simulink model "CDF_VSVPWM_Grid_Parallel"  (R2024b) (continued)
== system CDF_VSVPWM_Grid_Parallel/VSVPWM1/矢量作用时间计算/I3 ==
  note: Ta
  note: Tb
  note: Tc
  k : Inport
  Ts : Inport  Port=2
  theta : Inport  Port=3
  Constant : Constant
  Constant1 : Constant
  Gain : Gain  Gain=sqrt(3)
  Gain1 : Gain  Gain=2
  Gain3 : Gain  Gain=3
  Mux : Mux  Inputs=3
  Product{,1..5} (6 alike) : Product
  Scope{,1..3} (4 alike) : Scope
  Sin1 : Trigonometry  Operator=cos
  Sin2 : Trigonometry
  Sum2 : Sum  Inputs=|+-
  Sum3 : Sum  Inputs=|-+
  sin(pi/6+u) : MATLAB Function  TreatAsAtomicUnit=on
  T : Outport
  wires:
  Product5/1 -> Product2/1
  Gain3/1 -> Product5/2
  Sin2/1 -> Product5/1
  Sum3/1 -> Product1/1
  Constant1/1 -> Sum3/1
  Product4/1 -> Sum3/2
  Sin1/1 -> Product4/2
  Ts/1 -> Product/2, Product1/2, Product2/2
  Gain1/1 -> Product/1
  Sum2/1 -> Gain1/1
  Constant/1 -> Sum2/1
  Product3/1 -> Sum2/2
  sin(pi/6+u)/1(y) -> Product3/2
  Gain/1 -> Product3/1, Product4/1
  k/1 -> Gain/1, Gain3/1
  theta/1 -> Sin2/1, sin(pi/6+u)/1(u), Sin1/1
  Product1/1 -> Scope1/1, Mux/2
  Product/1 -> Scope/1, Mux/1
  Mux/1 -> Scope3/1, T/1
  Product2/1 -> Mux/3, Scope2/1

== system CDF_VSVPWM_Grid_Parallel/VSVPWM1/矢量作用时间计算/I4 ==
  note: Ta
  note: Tb
  note: Tc
  k : Inport
  Ts : Inport  Port=2
  theta : Inport  Port=3
  Constant : Constant
  Constant1 : Constant
  Gain : Gain  Gain=sqrt(3)
  Gain1 : Gain  Gain=2
  Gain3 : Gain  Gain=3
  Mux : Mux  Inputs=3
  Product{,1..5} (6 alike) : Product
  Scope{,1..3} (4 alike) : Scope
  Sin1 : Trigonometry  Operator=cos
  Sum2 : Sum  Inputs=|+-
  Sum3 : Sum  Inputs=|+-
  sin(pi/3-u) : MATLAB Function  TreatAsAtomicUnit=on
  sin(pi/6+u) : MATLAB Function  TreatAsAtomicUnit=on
  T : Outport
  wires:
  Sum3/1 -> Product1/1
  Gain1/1 -> Product/1
  Sum2/1 -> Gain1/1
  Constant/1 -> Sum2/1
  Product3/1 -> Sum2/2
  Gain/1 -> Product4/1, Product3/1
  k/1 -> Gain/1, Gain3/1
  theta/1 -> sin(pi/3-u)/1(u), sin(pi/6+u)/1(u), Sin1/1
  Product1/1 -> Scope2/1, Mux/2
  Product/1 -> Scope1/1, Mux/1
  Mux/1 -> Scope/1, T/1
  Product2/1 -> Mux/3, Scope3/1
  Sin1/1 -> Product3/2
  Ts/1 -> Product2/2, Product1/2, Product/2
  sin(pi/6+u)/1(y) -> Product4/2
  Constant1/1 -> Sum3/2
  Product4/1 -> Sum3/1
  sin(pi/3-u)/1(y) -> Product5/2
  Gain3/1 -> Product5/1
  Product5/1 -> Product2/1

== system CDF_VSVPWM_Grid_Parallel/VSVPWM1/矢量作用时间计算/I5 ==
  note: Tc
  note: Tb
  note: Ta
  k : Inport
  Ts : Inport  Port=2
  theta : Inport  Port=3
  Constant : Constant
  Constant1 : Constant
  Gain : Gain  Gain=sqrt(3)
  Gain1 : Gain  Gain=3
  Mux : Mux  Inputs=3
  Product{,1..5} (6 alike) : Product
  Scope : Scope
  Scope1 : Scope
  Scope2 : Scope
  Sin1 : Trigonometry  Operator=cos
  Sum1 : Sum  Inputs=|+-
  Sum2 : Sum  Inputs=|-+
  Sum3 : Sum  Inputs=|+-
  sin(pi/3+u) : MATLAB Function  TreatAsAtomicUnit=on
  sin(pi/6+u) : MATLAB Function  TreatAsAtomicUnit=on
  T : Outport
  wires:
  k/1 -> Product5/1, Gain/1
  sin(pi/3+u)/1(y) -> Product5/2
  Product4/1 -> Sum3/1
  Constant1/1 -> Sum1/1, Sum3/2
  sin(pi/6+u)/1(y) -> Product4/2
  Ts/1 -> Product/2, Product1/2, Product2/2
  Sin1/1 -> Product3/2
  Mux/1 -> T/1
  Product/1 -> Mux/1, Scope/1
  Product1/1 -> Scope1/1, Mux/2
  theta/1 -> Sin1/1, sin(pi/6+u)/1(u), sin(pi/3+u)/1(u)
  Gain/1 -> Product3/1, Product4/1
  Product3/1 -> Sum2/2
  Constant/1 -> Sum2/1
  Sum2/1 -> Product/1
  Sum3/1 -> Product1/1
  Product5/1 -> Sum1/2
  Sum1/1 -> Product2/1
  Product2/1 -> Gain1/1
  Gain1/1 -> Mux/3, Scope2/1

== system CDF_VSVPWM_Grid_Parallel/VSVPWM/生成时间调制波/Ⅰ扇区的调制信号计算/1-1 ==
  time : Inport
  Demux : Demux  Outputs=5
  MATLAB Function : MATLAB Function  TreatAsAtomicUnit=on
  MATLAB Function1 : MATLAB Function  TreatAsAtomicUnit=on
  MATLAB Function2 : MATLAB Function  TreatAsAtomicUnit=on
  Mux : Mux  Inputs=6
  Scope : Scope
  Tabc12 : Outport
  wires:
  MATLAB Function/1(ta1) -> Mux/1
  time/1 -> Demux/1
  Mux/1 -> Scope/1, Tabc12/1
  Demux/1 -> MATLAB Function2/1(unused), MATLAB Function1/1(T1), MATLAB Function/1(T1)
  MATLAB Function/2(ta2) -> Mux/2
  MATLAB Function1/1(tb1) -> Mux/3
  MATLAB Function1/2(tb2) -> Mux/4
  MATLAB Function2/1(tc1) -> Mux/5
  MATLAB Function2/2(tc2) -> Mux/6
  Demux/2 -> MATLAB Function2/2(unused1), MATLAB Function1/2(unused), MATLAB Function/2(T2)
  Demux/3 -> MATLAB Function2/3(unused2), MATLAB Function1/3(unused1), MATLAB Function/3(unused)
  Demux/4 -> MATLAB Function2/4(T4), MATLAB Function1/4(unused2), MATLAB Function/4(unused1)
  Demux/5 -> MATLAB Function2/5(T5), MATLAB Function1/5(T5), MATLAB Function/5(unused2)

== system CDF_VSVPWM_Grid_Parallel/VSVPWM/生成时间调制波/Ⅰ扇区的调制信号计算/1-2 ==
  time : Inport
  Demux : Demux  Outputs=5
  MATLAB Function : MATLAB Function  TreatAsAtomicUnit=on
  MATLAB Function1 : MATLAB Function  TreatAsAtomicUnit=on
  MATLAB Function2 : MATLAB Function  TreatAsAtomicUnit=on
  Mux : Mux  Inputs=6
  Tabc12 : Outport
  wires:
  MATLAB Function2/2(tc2) -> Mux/6
  MATLAB Function2/1(tc1) -> Mux/5
  MATLAB Function1/2(tb2) -> Mux/4
  MATLAB Function1/1(tb1) -> Mux/3
  MATLAB Function/2(ta2) -> Mux/2
  Demux/5 -> MATLAB Function/5(unused1), MATLAB Function1/5(T5), MATLAB Function2/5(T5)
  Demux/4 -> MATLAB Function/4(unused), MATLAB Function1/4(unused2), MATLAB Function2/4(T4)
  Demux/2 -> MATLAB Function/2(T2), MATLAB Function1/2(unused), MATLAB Function2/2(unused1)
  Demux/1 -> MATLAB Function/1(T1), MATLAB Function1/1(T1), MATLAB Function2/1(unused)
  Mux/1 -> Tabc12/1
  Demux/3 -> MATLAB Function/3(T3), MATLAB Function1/3(unused1), MATLAB Function2/3(T3)
  time/1 -> Demux/1
  MATLAB Function/1(ta1) -> Mux/1

== system CDF_VSVPWM_Grid_Parallel/VSVPWM/生成时间调制波/Ⅰ扇区的调制信号计算/1-3 ==
  time : Inport
  Demux : Demux  Outputs=5
  MATLAB Function : MATLAB Function  TreatAsAtomicUnit=on
  MATLAB Function1 : MATLAB Function  TreatAsAtomicUnit=on
  MATLAB Function2 : MATLAB Function  TreatAsAtomicUnit=on
  Mux : Mux  Inputs=6
  Tabc12 : Outport
  wires:
  MATLAB Function/1(ta1) -> Mux/1
  time/1 -> Demux/1
  Demux/3 -> MATLAB Function2/3(T3), MATLAB Function1/3(unused1), MATLAB Function/3(T3)
  Mux/1 -> Tabc12/1
  Demux/1 -> MATLAB Function2/1(unused), MATLAB Function1/1(T1), MATLAB Function/1(T1)
  Demux/2 -> MATLAB Function2/2(unused1), MATLAB Function1/2(unused), MATLAB Function/2(T2)
  Demux/4 -> MATLAB Function2/4(T4), MATLAB Function1/4(T4), MATLAB Function/4(T4)
  Demux/5 -> MATLAB Function2/5(T5), MATLAB Function1/5(T5), MATLAB Function/5(unused)
  MATLAB Function/2(ta2) -> Mux/2
  MATLAB Function1/1(tb1) -> Mux/3
  MATLAB Function1/2(tb2) -> Mux/4
  MATLAB Function2/1(tc1) -> Mux/5
  MATLAB Function2/2(tc2) -> Mux/6

== system CDF_VSVPWM_Grid_Parallel/VSVPWM/生成时间调制波/Ⅰ扇区的调制信号计算/1-4 ==
  time : Inport
  Demux : Demux  Outputs=5
  MATLAB Function : MATLAB Function  TreatAsAtomicUnit=on
  MATLAB Function1 : MATLAB Function  TreatAsAtomicUnit=on
  MATLAB Function2 : MATLAB Function  TreatAsAtomicUnit=on
  Mux : Mux  Inputs=6
  Tabc12 : Outport
  wires:
  MATLAB Function2/2(tc2) -> Mux/6
  MATLAB Function2/1(tc1) -> Mux/5
  MATLAB Function1/2(tb2) -> Mux/4
  MATLAB Function1/1(tb1) -> Mux/3
  MATLAB Function/2(ta2) -> Mux/2
  Demux/5 -> MATLAB Function/5(unused1), MATLAB Function1/5(T5), MATLAB Function2/5(T5)
  Demux/4 -> MATLAB Function/4(unused), MATLAB Function1/4(unused1), MATLAB Function2/4(T4)
  Demux/2 -> MATLAB Function/2(T2), MATLAB Function1/2(T2), MATLAB Function2/2(T2)
  Demux/1 -> MATLAB Function/1(T1), MATLAB Function1/1(T1), MATLAB Function2/1(unused)
  Mux/1 -> Tabc12/1
  Demux/3 -> MATLAB Function/3(T3), MATLAB Function1/3(unused), MATLAB Function2/3(T3)
  time/1 -> Demux/1
  MATLAB Function/1(ta1) -> Mux/1

== system CDF_VSVPWM_Grid_Parallel/VSVPWM/生成时间调制波/Ⅰ扇区的调制信号计算/1-5 ==
  time : Inport
  Demux : Demux  Outputs=5
  MATLAB Function : MATLAB Function  TreatAsAtomicUnit=on
  MATLAB Function1 : MATLAB Function  TreatAsAtomicUnit=on
  MATLAB Function2 : MATLAB Function  TreatAsAtomicUnit=on
  Mux : Mux  Inputs=6
  Tabc12 : Outport
  wires:
  MATLAB Function/1(ta1) -> Mux/1
  time/1 -> Demux/1
  Demux/3 -> MATLAB Function2/3(T3), MATLAB Function1/3(unused), MATLAB Function/3(T3)
  Mux/1 -> Tabc12/1
  Demux/1 -> MATLAB Function2/1(unused), MATLAB Function1/1(T1), MATLAB Function/1(T1)
  Demux/2 -> MATLAB Function2/2(T2), MATLAB Function1/2(T2), MATLAB Function/2(T2)
  Demux/4 -> MATLAB Function2/4(T4), MATLAB Function1/4(T4), MATLAB Function/4(T4)
  Demux/5 -> MATLAB Function2/5(T5), MATLAB Function1/5(T5), MATLAB Function/5(unused)
  MATLAB Function/2(ta2) -> Mux/2
  MATLAB Function1/1(tb1) -> Mux/3
  MATLAB Function1/2(tb2) -> Mux/4
  MATLAB Function2/1(tc1) -> Mux/5
  MATLAB Function2/2(tc2) -> Mux/6

== system CDF_VSVPWM_Grid_Parallel/VSVPWM/生成时间调制波/Ⅱ扇区的调制信号计算/2-1 ==
  time : Inport
  Demux : Demux  Outputs=5
  MATLAB Function : MATLAB Function  TreatAsAtomicUnit=on
  MATLAB Function1 : MATLAB Function  TreatAsAtomicUnit=on
  MATLAB Function2 : MATLAB Function  TreatAsAtomicUnit=on
  Mux : Mux  Inputs=6
  Scope : Scope  NumInputPorts=6
  Scope1 : Scope
  Tabc12 : Outport
  wires:
  MATLAB Function2/2(tc2) -> Scope/6, Mux/6
  MATLAB Function2/1(tc1) -> Scope/5, Mux/5
  MATLAB Function1/2(tb2) -> Scope/4, Mux/4
  MATLAB Function1/1(tb1) -> Scope/3, Mux/3
  MATLAB Function/2(ta2) -> Mux/2, Scope/2
  Demux/5 -> MATLAB Function/5(T5), MATLAB Function1/5(unused2), MATLAB Function2/5(T5)
  Demux/4 -> MATLAB Function/4(unused2), MATLAB Function1/4(unused1), MATLAB Function2/4(T4)
  Demux/2 -> MATLAB Function/2(unused), MATLAB Function1/2(T2), MATLAB Function2/2(unused1)
  Demux/1 -> MATLAB Function/1(T1), MATLAB Function1/1(T1), MATLAB Function2/1(unused)
  Mux/1 -> Scope1/1, Tabc12/1
  Demux/3 -> MATLAB Function/3(unused1), MATLAB Function1/3(unused), MATLAB Function2/3(unused2)
  time/1 -> Demux/1
  MATLAB Function/1(ta1) -> Scope/1, Mux/1

== system CDF_VSVPWM_Grid_Parallel/VSVPWM/生成时间调制波/Ⅱ扇区的调制信号计算/2-2 ==
  time : Inport
  Demux : Demux  Outputs=5
  MATLAB Function : MATLAB Function  TreatAsAtomicUnit=on
  MATLAB Function1 : MATLAB Function  TreatAsAtomicUnit=on
  MATLAB Function2 : MATLAB Function  TreatAsAtomicUnit=on
  Mux : Mux  Inputs=6
  Tabc12 : Outport
  wires:
  MATLAB Function/1(ta1) -> Mux/1
  time/1 -> Demux/1
  Demux/3 -> MATLAB Function2/3(T3), MATLAB Function1/3(T3), MATLAB Function/3(unused1)
  Mux/1 -> Tabc12/1
  Demux/1 -> MATLAB Function2/1(unused), MATLAB Function1/1(T1), MATLAB Function/1(T1)
  Demux/2 -> MATLAB Function2/2(unused1), MATLAB Function1/2(T2), MATLAB Function/2(unused)
  Demux/4 -> MATLAB Function2/4(T4), MATLAB Function1/4(unused), MATLAB Function/4(unused2)
  Demux/5 -> MATLAB Function2/5(T5), MATLAB Function1/5(unused1), MATLAB Function/5(T5)
  MATLAB Function/2(ta2) -> Mux/2
  MATLAB Function1/1(tb1) -> Mux/3
  MATLAB Function1/2(tb2) -> Mux/4
  MATLAB Function2/1(tc1) -> Mux/5
  MATLAB Function2/2(tc2) -> Mux/6

== system CDF_VSVPWM_Grid_Parallel/VSVPWM/生成时间调制波/Ⅱ扇区的调制信号计算/2-3 ==
  time : Inport
  Demux : Demux  Outputs=5
  MATLAB Function : MATLAB Function  TreatAsAtomicUnit=on
  MATLAB Function1 : MATLAB Function  TreatAsAtomicUnit=on
  MATLAB Function2 : MATLAB Function  TreatAsAtomicUnit=on
  Mux : Mux  Inputs=6
  Tabc12 : Outport
  wires:
  MATLAB Function2/2(tc2) -> Mux/6
  MATLAB Function2/1(tc1) -> Mux/5
  MATLAB Function1/2(tb2) -> Mux/4
  MATLAB Function1/1(tb1) -> Mux/3
  MATLAB Function/2(ta2) -> Mux/2
  Demux/5 -> MATLAB Function/5(T5), MATLAB Function1/5(unused1), MATLAB Function2/5(T5)
  Demux/4 -> MATLAB Function/4(unused1), MATLAB Function1/4(unused), MATLAB Function2/4(T4)
  Demux/2 -> MATLAB Function/2(T2), MATLAB Function1/2(T2), MATLAB Function2/2(T2)
  Demux/1 -> MATLAB Function/1(T1), MATLAB Function1/1(T1), MATLAB Function2/1(unused)
  Mux/1 -> Tabc12/1
  Demux/3 -> MATLAB Function/3(unused), MATLAB Function1/3(T3), MATLAB Function2/3(T3)
  time/1 -> Demux/1
  MATLAB Function/1(ta1) -> Mux/1

== system CDF_VSVPWM_Grid_Parallel/VSVPWM/生成时间调制波/Ⅱ扇区的调制信号计算/2-4 ==
  time : Inport
  Demux : Demux  Outputs=5
  MATLAB Function : MATLAB Function  TreatAsAtomicUnit=on
  MATLAB Function1 : MATLAB Function  TreatAsAtomicUnit=on
  MATLAB Function2 : MATLAB Function  TreatAsAtomicUnit=on
  Mux : Mux  Inputs=6
  Tabc12 : Outport
  wires:
  MATLAB Function/1(ta1) -> Mux/1
  time/1 -> Demux/1
  Demux/3 -> MATLAB Function2/3(T3), MATLAB Function1/3(T3), MATLAB Function/3(unused1)
  Mux/1 -> Tabc12/1
  Demux/1 -> MATLAB Function2/1(unused), MATLAB Function1/1(T1), MATLAB Function/1(T1)
  Demux/2 -> MATLAB Function2/2(unused1), MATLAB Function1/2(T2), MATLAB Function/2(unused)
  Demux/4 -> MATLAB Function2/4(T4), MATLAB Function1/4(T4), MATLAB Function/4(T4)
  Demux/5 -> MATLAB Function2/5(T5), MATLAB Function1/5(unused), MATLAB Function/5(T5)
  MATLAB Function/2(ta2) -> Mux/2
  MATLAB Function1/1(tb1) -> Mux/3
  MATLAB Function1/2(tb2) -> Mux/4
  MATLAB Function2/1(tc1) -> Mux/5
  MATLAB Function2/2(tc2) -> Mux/6

== system CDF_VSVPWM_Grid_Parallel/VSVPWM/生成时间调制波/Ⅱ扇区的调制信号计算/2-5 ==
  time : Inport
  Demux : Demux  Outputs=5
  MATLAB Function : MATLAB Function  TreatAsAtomicUnit=on
  MATLAB Function1 : MATLAB Function  TreatAsAtomicUnit=on
  MATLAB Function2 : MATLAB Function  TreatAsAtomicUnit=on
  Mux : Mux  Inputs=6
  Tabc12 : Outport
  wires:
  MATLAB Function2/2(tc2) -> Mux/6
  MATLAB Function2/1(tc1) -> Mux/5
  MATLAB Function1/2(tb2) -> Mux/4
  MATLAB Function1/1(tb1) -> Mux/3
  MATLAB Function/2(ta2) -> Mux/2
  Demux/5 -> MATLAB Function/5(T5), MATLAB Function1/5(unused), MATLAB Function2/5(T5)
  Demux/4 -> MATLAB Function/4(T4), MATLAB Function1/4(T4), MATLAB Function2/4(T4)
  Demux/2 -> MATLAB Function/2(T2), MATLAB Function1/2(T2), MATLAB Function2/2(T2)
  Demux/1 -> MATLAB Function/1(T1), MATLAB Function1/1(T1), MATLAB Function2/1(unused)
  Mux/1 -> Tabc12/1
  Demux/3 -> MATLAB Function/3(unused), MATLAB Function1/3(T3), MATLAB Function2/3(T3)
  time/1 -> Demux/1
  MATLAB Function/1(ta1) -> Mux/1

== system CDF_VSVPWM_Grid_Parallel/VSVPWM/生成时间调制波/Ⅲ扇区的调制信号计算1/1-1 ==
  time : Inport
  Demux : Demux  Outputs=5
  MATLAB Function : MATLAB Function  TreatAsAtomicUnit=on
  MATLAB Function1 : MATLAB Function  TreatAsAtomicUnit=on
  MATLAB Function2 : MATLAB Function  TreatAsAtomicUnit=on
  Mux : Mux  Inputs=6
  Scope : Scope  NumInputPorts=6
  Scope1 : Scope
  Tabc12 : Outport
  wires:
  Demux/5 -> MATLAB Function/5(unused2), MATLAB Function1/5(T5), MATLAB Function2/5(T5)
  Demux/4 -> MATLAB Function/4(unused1), MATLAB Function1/4(unused2), MATLAB Function2/4(T4)
  Demux/3 -> MATLAB Function/3(unused), MATLAB Function1/3(unused1), MATLAB Function2/3(unused2)
  Demux/2 -> MATLAB Function/2(T2), MATLAB Function1/2(unused), MATLAB Function2/2(unused1)
  Demux/1 -> MATLAB Function/1(T1), MATLAB Function1/1(T1), MATLAB Function2/1(unused)
  Mux/1 -> Scope1/1, Tabc12/1
  time/1 -> Demux/1
  MATLAB Function/1(ta1) -> Scope/1, Mux/3
  MATLAB Function/2(ta2) -> Mux/4, Scope/2
  MATLAB Function1/1(tb1) -> Scope/3, Mux/5
  MATLAB Function1/2(tb2) -> Mux/6, Scope/4
  MATLAB Function2/1(tc1) -> Scope/5, Mux/1
  MATLAB Function2/2(tc2) -> Scope/6, Mux/2

== system CDF_VSVPWM_Grid_Parallel/VSVPWM/生成时间调制波/Ⅲ扇区的调制信号计算1/1-2 ==
  time : Inport
  Demux : Demux  Outputs=5
  MATLAB Function : MATLAB Function  TreatAsAtomicUnit=on
  MATLAB Function1 : MATLAB Function  TreatAsAtomicUnit=on
  MATLAB Function2 : MATLAB Function  TreatAsAtomicUnit=on
  Mux : Mux  Inputs=6
  Tabc12 : Outport
  wires:
  time/1 -> Demux/1
  Demux/3 -> MATLAB Function2/3(T3), MATLAB Function1/3(unused1), MATLAB Function/3(T3)
  Mux/1 -> Tabc12/1
  Demux/1 -> MATLAB Function2/1(unused), MATLAB Function1/1(T1), MATLAB Function/1(T1)
  Demux/2 -> MATLAB Function2/2(unused1), MATLAB Function1/2(unused), MATLAB Function/2(T2)
  Demux/4 -> MATLAB Function2/4(T4), MATLAB Function1/4(unused2), MATLAB Function/4(unused)
  Demux/5 -> MATLAB Function2/5(T5), MATLAB Function1/5(T5), MATLAB Function/5(unused1)
  MATLAB Function/1(ta1) -> Mux/3
  MATLAB Function/2(ta2) -> Mux/4
  MATLAB Function1/1(tb1) -> Mux/5
  MATLAB Function1/2(tb2) -> Mux/6
  MATLAB Function2/1(tc1) -> Mux/1
  MATLAB Function2/2(tc2) -> Mux/2

== system CDF_VSVPWM_Grid_Parallel/VSVPWM/生成时间调制波/Ⅲ扇区的调制信号计算1/1-3 ==
  time : Inport
  Demux : Demux  Outputs=5
  MATLAB Function : MATLAB Function  TreatAsAtomicUnit=on
  MATLAB Function1 : MATLAB Function  TreatAsAtomicUnit=on
  MATLAB Function2 : MATLAB Function  TreatAsAtomicUnit=on
  Mux : Mux  Inputs=6
  Scope1 : Scope
  Tabc12 : Outport
  wires:
  Demux/5 -> MATLAB Function/5(unused), MATLAB Function1/5(T5), MATLAB Function2/5(T5)
  Demux/4 -> MATLAB Function/4(T4), MATLAB Function1/4(T4), MATLAB Function2/4(T4)
  Demux/2 -> MATLAB Function/2(T2), MATLAB Function1/2(unused), MATLAB Function2/2(unused1)
  Demux/1 -> MATLAB Function/1(T1), MATLAB Function1/1(T1), MATLAB Function2/1(unused)
  Mux/1 -> Scope1/1, Tabc12/1
  Demux/3 -> MATLAB Function/3(T3), MATLAB Function1/3(unused1), MATLAB Function2/3(T3)
  time/1 -> Demux/1
  MATLAB Function/1(ta1) -> Mux/3
  MATLAB Function/2(ta2) -> Mux/4
  MATLAB Function1/1(tb1) -> Mux/5
  MATLAB Function1/2(tb2) -> Mux/6
  MATLAB Function2/1(tc1) -> Mux/1
  MATLAB Function2/2(tc2) -> Mux/2

== system CDF_VSVPWM_Grid_Parallel/VSVPWM/生成时间调制波/Ⅲ扇区的调制信号计算1/1-4 ==
  time : Inport
  Demux : Demux  Outputs=5
  MATLAB Function : MATLAB Function  TreatAsAtomicUnit=on
  MATLAB Function1 : MATLAB Function  TreatAsAtomicUnit=on
  MATLAB Function2 : MATLAB Function  TreatAsAtomicUnit=on
  Mux : Mux  Inputs=6
  Tabc12 : Outport
  wires:
  time/1 -> Demux/1
  Demux/3 -> MATLAB Function2/3(T3), MATLAB Function1/3(unused), MATLAB Function/3(T3)
  Mux/1 -> Tabc12/1
  Demux/1 -> MATLAB Function2/1(unused), MATLAB Function1/1(T1), MATLAB Function/1(T1)
  Demux/2 -> MATLAB Function2/2(T2), MATLAB Function1/2(T2), MATLAB Function/2(T2)
  Demux/4 -> MATLAB Function2/4(T4), MATLAB Function1/4(unused1), MATLAB Function/4(unused)
  Demux/5 -> MATLAB Function2/5(T5), MATLAB Function1/5(T5), MATLAB Function/5(unused1)
  MATLAB Function/1(ta1) -> Mux/3
  MATLAB Function/2(ta2) -> Mux/4
  MATLAB Function1/1(tb1) -> Mux/5
  MATLAB Function1/2(tb2) -> Mux/6
  MATLAB Function2/1(tc1) -> Mux/1
  MATLAB Function2/2(tc2) -> Mux/2

== system CDF_VSVPWM_Grid_Parallel/VSVPWM/生成时间调制波/Ⅲ扇区的调制信号计算1/1-5 ==
  time : Inport
  Demux : Demux  Outputs=5
  MATLAB Function : MATLAB Function  TreatAsAtomicUnit=on
  MATLAB Function1 : MATLAB Function  TreatAsAtomicUnit=on
  MATLAB Function2 : MATLAB Function  TreatAsAtomicUnit=on
  Mux : Mux  Inputs=6
  Tabc12 : Outport
  wires:
  Demux/5 -> MATLAB Function/5(unused), MATLAB Function1/5(T5), MATLAB Function2/5(T5)
  Demux/4 -> MATLAB Function/4(T4), MATLAB Function1/4(T4), MATLAB Function2/4(T4)
  Demux/2 -> MATLAB Function/2(T2), MATLAB Function1/2(T2), MATLAB Function2/2(T2)
  Demux/1 -> MATLAB Function/1(T1), MATLAB Function1/1(T1), MATLAB Function2/1(unused)
  Mux/1 -> Tabc12/1
  Demux/3 -> MATLAB Function/3(T3), MATLAB Function1/3(unused), MATLAB Function2/3(T3)
  time/1 -> Demux/1
  MATLAB Function/1(ta1) -> Mux/3
  MATLAB Function/2(ta2) -> Mux/4
  MATLAB Function1/1(tb1) -> Mux/5
  MATLAB Function1/2(tb2) -> Mux/6
  MATLAB Function2/1(tc1) -> Mux/1
  MATLAB Function2/2(tc2) -> Mux/2

== system CDF_VSVPWM_Grid_Parallel/VSVPWM/生成时间调制波/Ⅳ扇区的调制信号计算/2-1 ==
  time : Inport
  Demux : Demux  Outputs=5
  MATLAB Function : MATLAB Function  TreatAsAtomicUnit=on
  MATLAB Function1 : MATLAB Function  TreatAsAtomicUnit=on
  MATLAB Function2 : MATLAB Function  TreatAsAtomicUnit=on
  Mux : Mux  Inputs=6
  Scope : Scope
  Tabc12 : Outport
  wires:
  time/1 -> Demux/1
  Demux/3 -> MATLAB Function2/3(unused2), MATLAB Function1/3(unused), MATLAB Function/3(unused1)
  Demux/1 -> MATLAB Function2/1(unused), MATLAB Function1/1(T1), MATLAB Function/1(T1)
  Demux/2 -> MATLAB Function2/2(unused1), MATLAB Function1/2(T2), MATLAB Function/2(unused)
  Demux/4 -> MATLAB Function2/4(T4), MATLAB Function1/4(unused1), MATLAB Function/4(unused2)
  Demux/5 -> MATLAB Function2/5(T5), MATLAB Function1/5(unused2), MATLAB Function/5(T5)
  MATLAB Function/2(ta2) -> Mux/4
  MATLAB Function2/1(tc1) -> Mux/1
  MATLAB Function/1(ta1) -> Mux/3
  MATLAB Function2/2(tc2) -> Mux/2
  MATLAB Function1/2(tb2) -> Mux/6
  Mux/1 -> Scope/1, Tabc12/1
  MATLAB Function1/1(tb1) -> Mux/5

== system CDF_VSVPWM_Grid_Parallel/VSVPWM/生成时间调制波/Ⅳ扇区的调制信号计算/2-2 ==
  time : Inport
  Demux : Demux  Outputs=5
  MATLAB Function : MATLAB Function  TreatAsAtomicUnit=on
  MATLAB Function1 : MATLAB Function  TreatAsAtomicUnit=on
  MATLAB Function2 : MATLAB Function  TreatAsAtomicUnit=on
  Mux : Mux  Inputs=6
  Tabc12 : Outport
  wires:
  Demux/5 -> MATLAB Function/5(T5), MATLAB Function1/5(unused1), MATLAB Function2/5(T5)
  Demux/4 -> MATLAB Function/4(unused2), MATLAB Function1/4(unused), MATLAB Function2/4(T4)
  Demux/2 -> MATLAB Function/2(unused), MATLAB Function1/2(T2), MATLAB Function2/2(unused1)
  Demux/1 -> MATLAB Function/1(T1), MATLAB Function1/1(T1), MATLAB Function2/1(unused)
  Demux/3 -> MATLAB Function/3(unused1), MATLAB Function1/3(T3), MATLAB Function2/3(T3)
  time/1 -> Demux/1
  MATLAB Function1/2(tb2) -> Mux/6
  MATLAB Function/1(ta1) -> Mux/3
  MATLAB Function2/1(tc1) -> Mux/1
  MATLAB Function1/1(tb1) -> Mux/5
  Mux/1 -> Tabc12/1
  MATLAB Function/2(ta2) -> Mux/4
  MATLAB Function2/2(tc2) -> Mux/2

== system CDF_VSVPWM_Grid_Parallel/VSVPWM/生成时间调制波/Ⅳ扇区的调制信号计算/2-3 ==
  time : Inport
  Demux : Demux  Outputs=5
  MATLAB Function : MATLAB Function  TreatAsAtomicUnit=on
  MATLAB Function1 : MATLAB Function  TreatAsAtomicUnit=on
  MATLAB Function2 : MATLAB Function  TreatAsAtomicUnit=on
  Mux : Mux  Inputs=6
  Tabc12 : Outport
  wires:
  time/1 -> Demux/1
  Demux/3 -> MATLAB Function2/3(T3), MATLAB Function1/3(T3), MATLAB Function/3(unused)
  Demux/1 -> MATLAB Function2/1(unused), MATLAB Function1/1(T1), MATLAB Function/1(T1)
  Demux/2 -> MATLAB Function2/2(T2), MATLAB Function1/2(T2), MATLAB Function/2(T2)
  Demux/4 -> MATLAB Function2/4(T4), MATLAB Function1/4(unused), MATLAB Function/4(unused1)
  Demux/5 -> MATLAB Function2/5(T5), MATLAB Function1/5(unused1), MATLAB Function/5(T5)
  MATLAB Function1/2(tb2) -> Mux/6
  MATLAB Function/1(ta1) -> Mux/3
  MATLAB Function2/1(tc1) -> Mux/1
  MATLAB Function1/1(tb1) -> Mux/5
  Mux/1 -> Tabc12/1
  MATLAB Function/2(ta2) -> Mux/4
  MATLAB Function2/2(tc2) -> Mux/2

== system CDF_VSVPWM_Grid_Parallel/VSVPWM/生成时间调制波/Ⅳ扇区的调制信号计算/2-4 ==
  time : Inport
  Demux : Demux  Outputs=5
  MATLAB Function : MATLAB Function  TreatAsAtomicUnit=on
  MATLAB Function1 : MATLAB Function  TreatAsAtomicUnit=on
  MATLAB Function2 : MATLAB Function  TreatAsAtomicUnit=on
  Mux : Mux  Inputs=6
  Tabc12 : Outport
  wires:
  Demux/5 -> MATLAB Function/5(T5), MATLAB Function1/5(unused), MATLAB Function2/5(T5)
  Demux/4 -> MATLAB Function/4(T4), MATLAB Function1/4(T4), MATLAB Function2/4(T4)
  Demux/2 -> MATLAB Function/2(unused), MATLAB Function1/2(T2), MATLAB Function2/2(unused1)
  Demux/1 -> MATLAB Function/1(T1), MATLAB Function1/1(T1), MATLAB Function2/1(unused)
  Demux/3 -> MATLAB Function/3(unused1), MATLAB Function1/3(T3), MATLAB Function2/3(T3)
  time/1 -> Demux/1
  MATLAB Function1/2(tb2) -> Mux/6
  MATLAB Function/1(ta1) -> Mux/3
  MATLAB Function2/1(tc1) -> Mux/1
  MATLAB Function1/1(tb1) -> Mux/5
  Mux/1 -> Tabc12/1
  MATLAB Function/2(ta2) -> Mux/4
  MATLAB Function2/2(tc2) -> Mux/2

== system CDF_VSVPWM_Grid_Parallel/VSVPWM/生成时间调制波/Ⅳ扇区的调制信号计算/2-5 ==
  time : Inport
  Demux : Demux  Outputs=5
  MATLAB Function : MATLAB Function  TreatAsAtomicUnit=on
  MATLAB Function1 : MATLAB Function  TreatAsAtomicUnit=on
  MATLAB Function2 : MATLAB Function  TreatAsAtomicUnit=on
  Mux : Mux  Inputs=6
  Tabc12 : Outport
  wires:
  time/1 -> Demux/1
  Demux/3 -> MATLAB Function2/3(T3), MATLAB Function1/3(T3), MATLAB Function/3(unused)
  Demux/1 -> MATLAB Function2/1(unused), MATLAB Function1/1(T1), MATLAB Function/1(T1)
  Demux/2 -> MATLAB Function2/2(T2), MATLAB Function1/2(T2), MATLAB Function/2(T2)
  Demux/4 -> MATLAB Function2/4(T4), MATLAB Function1/4(T4), MATLAB Function/4(T4)
  Demux/5 -> MATLAB Function2/5(T5), MATLAB Function1/5(unused), MATLAB Function/5(T5)
  MATLAB Function1/2(tb2) -> Mux/6
  MATLAB Function/1(ta1) -> Mux/3
  MATLAB Function2/1(tc1) -> Mux/1
  MATLAB Function1/1(tb1) -> Mux/5
  Mux/1 -> Tabc12/1
  MATLAB Function/2(ta2) -> Mux/4
  MATLAB Function2/2(tc2) -> Mux/2

== system CDF_VSVPWM_Grid_Parallel/VSVPWM/生成时间调制波/Ⅴ扇区的调制信号计算/1-1 ==
  time : Inport
  Demux : Demux  Outputs=5
  MATLAB Function : MATLAB Function  TreatAsAtomicUnit=on
  MATLAB Function1 : MATLAB Function  TreatAsAtomicUnit=on
  MATLAB Function2 : MATLAB Function  TreatAsAtomicUnit=on
  Mux : Mux  Inputs=6
  Scope : Scope
  Tabc12 : Outport
  wires:
  Demux/5 -> MATLAB Function/5(unused2), MATLAB Function1/5(T5), MATLAB Function2/5(T5)
  Demux/4 -> MATLAB Function/4(unused1), MATLAB Function1/4(unused2), MATLAB Function2/4(T4)
  Demux/3 -> MATLAB Function/3(unused), MATLAB Function1/3(unused1), MATLAB Function2/3(unused2)
  Demux/2 -> MATLAB Function/2(T2), MATLAB Function1/2(unused), MATLAB Function2/2(unused1)
  Demux/1 -> MATLAB Function/1(T1), MATLAB Function1/1(T1), MATLAB Function2/1(unused)
  Mux/1 -> Scope/1, Tabc12/1
  time/1 -> Demux/1
  MATLAB Function/1(ta1) -> Mux/5
  MATLAB Function/2(ta2) -> Mux/6
  MATLAB Function1/1(tb1) -> Mux/1
  MATLAB Function1/2(tb2) -> Mux/2
  MATLAB Function2/1(tc1) -> Mux/3
  MATLAB Function2/2(tc2) -> Mux/4

== system CDF_VSVPWM_Grid_Parallel/VSVPWM/生成时间调制波/Ⅴ扇区的调制信号计算/1-2 ==
  time : Inport
  Demux : Demux  Outputs=5
  MATLAB Function : MATLAB Function  TreatAsAtomicUnit=on
  MATLAB Function1 : MATLAB Function  TreatAsAtomicUnit=on
  MATLAB Function2 : MATLAB Function  TreatAsAtomicUnit=on
  Mux : Mux  Inputs=6
  Tabc12 : Outport
  wires:
  time/1 -> Demux/1
  Demux/3 -> MATLAB Function2/3(T3), MATLAB Function1/3(unused1), MATLAB Function/3(T3)
  Mux/1 -> Tabc12/1
  Demux/1 -> MATLAB Function2/1(unused), MATLAB Function1/1(T1), MATLAB Function/1(T1)
  Demux/2 -> MATLAB Function2/2(unused1), MATLAB Function1/2(unused), MATLAB Function/2(T2)
  Demux/4 -> MATLAB Function2/4(T4), MATLAB Function1/4(unused2), MATLAB Function/4(unused)
  Demux/5 -> MATLAB Function2/5(T5), MATLAB Function1/5(T5), MATLAB Function/5(unused1)
  MATLAB Function/1(ta1) -> Mux/5
  MATLAB Function/2(ta2) -> Mux/6
  MATLAB Function1/1(tb1) -> Mux/1
  MATLAB Function1/2(tb2) -> Mux/2
  MATLAB Function2/1(tc1) -> Mux/3
  MATLAB Function2/2(tc2) -> Mux/4

== system CDF_VSVPWM_Grid_Parallel/VSVPWM/生成时间调制波/Ⅴ扇区的调制信号计算/1-3 ==
  time : Inport
  Demux : Demux  Outputs=5
  MATLAB Function : MATLAB Function  TreatAsAtomicUnit=on
  MATLAB Function1 : MATLAB Function  TreatAsAtomicUnit=on
  MATLAB Function2 : MATLAB Function  TreatAsAtomicUnit=on
  Mux : Mux  Inputs=6
  Tabc12 : Outport
  wires:
  Demux/5 -> MATLAB Function/5(unused), MATLAB Function1/5(T5), MATLAB Function2/5(T5)
  Demux/4 -> MATLAB Function/4(T4), MATLAB Function1/4(T4), MATLAB Function2/4(T4)
  Demux/2 -> MATLAB Function/2(T2), MATLAB Function1/2(unused), MATLAB Function2/2(unused1)
  Demux/1 -> MATLAB Function/1(T1), MATLAB Function1/1(T1), MATLAB Function2/1(unused)
  Mux/1 -> Tabc12/1
  Demux/3 -> MATLAB Function/3(T3), MATLAB Function1/3(unused1), MATLAB Function2/3(T3)
  time/1 -> Demux/1
  MATLAB Function/1(ta1) -> Mux/5
  MATLAB Function/2(ta2) -> Mux/6
  MATLAB Function1/1(tb1) -> Mux/1
  MATLAB Function1/2(tb2) -> Mux/2
  MATLAB Function2/1(tc1) -> Mux/3
  MATLAB Function2/2(tc2) -> Mux/4
